simulink model "dss_2in_2048ch_983mhz_real"  (R2021a) (continued)
== system dss_2in_2048ch_983mhz_real == (continued)
      mask DialogControl t230_QT_dac3_AnalogSettings Prompt: Analog Settings
      mask DialogControl t230_DualTileConfig Prompt: Dual Tile Configuration
      mask DialogControl t230_DT_dac0 Prompt: DAC 0
      mask DialogControl t230_DT_dac0_DataSettings Prompt: Data Settings
      mask DialogControl t230_DT_dac0_req_axis_clk_label Prompt: Required AXI4-Stream clock:
      mask DialogControl t230_DT_dac0_MixerSettings Prompt: MIxer Settings
      mask DialogControl t230_DT_dac0_AnalogSettings Prompt: Analog Settings
      mask DialogControl t230_DT_dac1 Prompt: DAC 1
      mask DialogControl t230_DT_dac1_DataSettings Prompt: Data Settings
      mask DialogControl t230_DT_dac1_req_axis_clk_label Prompt: Required AXI4-Stream clock:
      mask DialogControl t230_DT_dac1_MixerSettings Prompt: MIxer Settings
      mask DialogControl t230_DT_dac1_AnalogSettings Prompt: Analog Settings
      mask DialogControl Tile231_container Prompt: Tile 231
      mask DialogControl pll_clocking_config_231 Prompt: Clocking and PLL Configuration
      mask DialogControl t231_QuadTileConfig Prompt: Quad Tile Configuration
      mask DialogControl t231_DACPair01 Prompt: DAC Pair 0,1
      mask DialogControl t231_QT_dac0 Prompt: DAC 0
      mask DialogControl t231_QT_dac0_DataSettings Prompt: Data Settings
      mask DialogControl t231_QT_dac0_req_axis_clk_label Prompt: Required AXI4-Stream clock:
      mask DialogControl t231_QT_dac0_MixerSettings Prompt: MIxer Settings
      mask DialogControl t231_QT_dac0_AnalogSettings Prompt: Analog Settings
      mask DialogControl t231_QT_dac1 Prompt: DAC 1
      mask DialogControl t231_QT_dac1_DataSettings Prompt: Data Settings
      mask DialogControl t231_QT_dac1_req_axis_clk_label Prompt: Required AXI4-Stream clock:
      mask DialogControl t231_QT_dac1_MixerSettings Prompt: MIxer Settings
      mask DialogControl t231_QT_dac1_AnalogSettings Prompt: Analog Settings
      mask DialogControl t231_DACPair23 Prompt: DAC Pair 2,3
      mask DialogControl t231_QT_dac2 Prompt: DAC 2
      mask DialogControl t231_QT_dac2_DataSettings Prompt: Data Settings
      mask DialogControl t231_QT_dac2_req_axis_clk_label Prompt: Required AXI4-Stream clock:
      mask DialogControl t231_QT_dac2_MixerSettings Prompt: MIxer Settings
      mask DialogControl t231_QT_dac2_AnalogSettings Prompt: Analog Settings
      mask DialogControl t231_QT_dac3 Prompt: DAC 3
      mask DialogControl t231_QT_dac3_DataSettings Prompt: Data Settings
      mask DialogControl t231_QT_dac3_req_axis_clk_label Prompt: Required AXI4-Stream clock:
      mask DialogControl t231_QT_dac3_MixerSettings Prompt: MIxer Settings
      mask DialogControl t231_QT_dac3_AnalogSettings Prompt: Analog Settings
      mask DialogControl t231_DualTileConfig Prompt: Dual Tile Configuration
      mask DialogControl t231_DT_dac0 Prompt: DAC 0
      mask DialogControl t231_DT_dac0_DataSettings Prompt: Data Settings
      mask DialogControl t231_DT_dac0_req_axis_clk_label Prompt: Required AXI4-Stream clock:
      mask DialogControl t231_DT_dac0_MixerSettings Prompt: MIxer Settings
      mask DialogControl t231_DT_dac0_AnalogSettings Prompt: Analog Settings
      mask DialogControl t231_DT_dac1 Prompt: DAC 1
      mask DialogControl t231_DT_dac1_DataSettings Prompt: Data Settings
      mask DialogControl t231_DT_dac1_req_axis_clk_label Prompt: Required AXI4-Stream clock:
      mask DialogControl t231_DT_dac1_MixerSettings Prompt: MIxer Settings
      mask DialogControl t231_DT_dac1_AnalogSettings Prompt: Analog Settings
      mask DialogControl SystemClocking Prompt: System Clocking
      mask DialogControl ADCClocking Prompt: ADC Tile Clocking
      mask DialogControl t224_clock_config Prompt: ADC Tile 224
      mask DialogControl t225_clock_config Prompt: ADC Tile 225
      mask DialogControl t226_clock_config Prompt: ADC Tile 226
      mask DialogControl t227_clock_config Prompt: ADC Tile 227
      mask DialogControl DACClocking Prompt: DAC Tile Clocking
      mask DialogControl t228_clock_config Prompt: DAC Tile 228
      mask DialogControl t229_clock_config Prompt: DAC Tile 229
      mask DialogControl t230_clock_config Prompt: DAC Tile 230
      mask DialogControl t231_clock_config Prompt: DAC Tile 231
  simple_bram_vacc : Subsystem  AncestorBlock=casper_library_accumulators/simple_bram_vacc  mask(simple_bram_vacc){vec_len=512, arith_type=Unsigned, n_bits=64, bin_pt=34}
      mask prompts: vec_len "Vector length:"; arith_type "Output type:"; n_bits "Bit width (output):"; bin_pt "Binary point (output):"
      mask help: This simple vector accumulator is based on a shift register. It outputs its previous accumulated vector after a new_acc pulse is received.
      mask init:
      | simple_bram_vacc_init(gcb, ...
      |     'vec_len', vec_len, ...
      |     'arith_type', arith_type, ...
      |     'n_bits', n_bits, ...
      |     'bin_pt', bin_pt);
      mask TypeOptions arith_type: Signed | Unsigned
  simple_bram_vacc1 : Subsystem  AncestorBlock=casper_library_accumulators/simple_bram_vacc  mask(simple_bram_vacc){vec_len=512, arith_type=Unsigned, n_bits=64, bin_pt=34}
      mask prompts: vec_len "Vector length:"; arith_type "Output type:"; n_bits "Bit width (output):"; bin_pt "Binary point (output):"
      mask help: This simple vector accumulator is based on a shift register. It outputs its previous accumulated vector after a new_acc pulse is received.
      mask init:
      | simple_bram_vacc_init(gcb, ...
      |     'vec_len', vec_len, ...
      |     'arith_type', arith_type, ...
      |     'n_bits', n_bits, ...
      |     'bin_pt', bin_pt);
      mask TypeOptions arith_type: Signed | Unsigned
  simple_bram_vacc10 : Subsystem  AncestorBlock=casper_library_accumulators/simple_bram_vacc  mask(simple_bram_vacc){vec_len=512, arith_type=Signed, n_bits=64, bin_pt=34}
      mask prompts: vec_len "Vector length:"; arith_type "Output type:"; n_bits "Bit width (output):"; bin_pt "Binary point (output):"
      mask help: This simple vector accumulator is based on a shift register. It outputs its previous accumulated vector after a new_acc pulse is received.
      mask init:
      | simple_bram_vacc_init(gcb, ...
      |     'vec_len', vec_len, ...
      |     'arith_type', arith_type, ...
      |     'n_bits', n_bits, ...
      |     'bin_pt', bin_pt);
      mask TypeOptions arith_type: Signed | Unsigned
  simple_bram_vacc11 : Subsystem  AncestorBlock=casper_library_accumulators/simple_bram_vacc  mask(simple_bram_vacc){vec_len=512, arith_type=Signed, n_bits=64, bin_pt=34}
      mask prompts: vec_len "Vector length:"; arith_type "Output type:"; n_bits "Bit width (output):"; bin_pt "Binary point (output):"
      mask help: This simple vector accumulator is based on a shift register. It outputs its previous accumulated vector after a new_acc pulse is received.
      mask init:
      | simple_bram_vacc_init(gcb, ...
      |     'vec_len', vec_len, ...
      |     'arith_type', arith_type, ...
      |     'n_bits', n_bits, ...
      |     'bin_pt', bin_pt);
      mask TypeOptions arith_type: Signed | Unsigned
  simple_bram_vacc12 : Subsystem  AncestorBlock=casper_library_accumulators/simple_bram_vacc  mask(simple_bram_vacc){vec_len=512, arith_type=Signed, n_bits=64, bin_pt=34}
      mask prompts: vec_len "Vector length:"; arith_type "Output type:"; n_bits "Bit width (output):"; bin_pt "Binary point (output):"
      mask help: This simple vector accumulator is based on a shift register. It outputs its previous accumulated vector after a new_acc pulse is received.
      mask init:
      | simple_bram_vacc_init(gcb, ...
      |     'vec_len', vec_len, ...
      |     'arith_type', arith_type, ...
      |     'n_bits', n_bits, ...
      |     'bin_pt', bin_pt);
      mask TypeOptions arith_type: Signed | Unsigned
  simple_bram_vacc13 : Subsystem  AncestorBlock=casper_library_accumulators/simple_bram_vacc  mask(simple_bram_vacc){vec_len=512, arith_type=Signed, n_bits=64, bin_pt=34}
      mask prompts: vec_len "Vector length:"; arith_type "Output type:"; n_bits "Bit width (output):"; bin_pt "Binary point (output):"
      mask help: This simple vector accumulator is based on a shift register. It outputs its previous accumulated vector after a new_acc pulse is received.
      mask init:
      | simple_bram_vacc_init(gcb, ...
      |     'vec_len', vec_len, ...
      |     'arith_type', arith_type, ...
      |     'n_bits', n_bits, ...
      |     'bin_pt', bin_pt);
      mask TypeOptions arith_type: Signed | Unsigned
  simple_bram_vacc14 : Subsystem  AncestorBlock=casper_library_accumulators/simple_bram_vacc  mask(simple_bram_vacc){vec_len=512, arith_type=Signed, n_bits=64, bin_pt=34}
      mask prompts: vec_len "Vector length:"; arith_type "Output type:"; n_bits "Bit width (output):"; bin_pt "Binary point (output):"
      mask help: This simple vector accumulator is based on a shift register. It outputs its previous accumulated vector after a new_acc pulse is received.
      mask init:
      | simple_bram_vacc_init(gcb, ...
      |     'vec_len', vec_len, ...
      |     'arith_type', arith_type, ...
      |     'n_bits', n_bits, ...
      |     'bin_pt', bin_pt);
      mask TypeOptions arith_type: Signed | Unsigned
  simple_bram_vacc15 : Subsystem  AncestorBlock=casper_library_accumulators/simple_bram_vacc  mask(simple_bram_vacc){vec_len=512, arith_type=Signed, n_bits=64, bin_pt=34}
      mask prompts: vec_len "Vector length:"; arith_type "Output type:"; n_bits "Bit width (output):"; bin_pt "Binary point (output):"
      mask help: This simple vector accumulator is based on a shift register. It outputs its previous accumulated vector after a new_acc pulse is received.
      mask init:
      | simple_bram_vacc_init(gcb, ...
      |     'vec_len', vec_len, ...
      |     'arith_type', arith_type, ...
      |     'n_bits', n_bits, ...
      |     'bin_pt', bin_pt);
      mask TypeOptions arith_type: Signed | Unsigned
  simple_bram_vacc16 : Subsystem  AncestorBlock=casper_library_accumulators/simple_bram_vacc  mask(simple_bram_vacc){vec_len=512, arith_type=Unsigned, n_bits=64, bin_pt=34}
      mask prompts: vec_len "Vector length:"; arith_type "Output type:"; n_bits "Bit width (output):"; bin_pt "Binary point (output):"
      mask help: This simple vector accumulator is based on a shift register. It outputs its previous accumulated vector after a new_acc pulse is received.
      mask init:
      | simple_bram_vacc_init(gcb, ...
      |     'vec_len', vec_len, ...
      |     'arith_type', arith_type, ...
      |     'n_bits', n_bits, ...
      |     'bin_pt', bin_pt);
      mask TypeOptions arith_type: Signed | Unsigned
  simple_bram_vacc17 : Subsystem  AncestorBlock=casper_library_accumulators/simple_bram_vacc  mask(simple_bram_vacc){vec_len=512, arith_type=Unsigned, n_bits=64, bin_pt=34}
      mask prompts: vec_len "Vector length:"; arith_type "Output type:"; n_bits "Bit width (output):"; bin_pt "Binary point (output):"
      mask help: This simple vector accumulator is based on a shift register. It outputs its previous accumulated vector after a new_acc pulse is received.
      mask init:
      | simple_bram_vacc_init(gcb, ...
      |     'vec_len', vec_len, ...
      |     'arith_type', arith_type, ...
      |     'n_bits', n_bits, ...
      |     'bin_pt', bin_pt);
      mask TypeOptions arith_type: Signed | Unsigned
  simple_bram_vacc18 : Subsystem  AncestorBlock=casper_library_accumulators/simple_bram_vacc  mask(simple_bram_vacc){vec_len=512, arith_type=Unsigned, n_bits=64, bin_pt=34}
      mask prompts: vec_len "Vector length:"; arith_type "Output type:"; n_bits "Bit width (output):"; bin_pt "Binary point (output):"
      mask help: This simple vector accumulator is based on a shift register. It outputs its previous accumulated vector after a new_acc pulse is received.
      mask init:
      | simple_bram_vacc_init(gcb, ...
      |     'vec_len', vec_len, ...
      |     'arith_type', arith_type, ...
      |     'n_bits', n_bits, ...
      |     'bin_pt', bin_pt);
      mask TypeOptions arith_type: Signed | Unsigned
  simple_bram_vacc19 : Subsystem  AncestorBlock=casper_library_accumulators/simple_bram_vacc  mask(simple_bram_vacc){vec_len=512, arith_type=Unsigned, n_bits=64, bin_pt=34}
      mask prompts: vec_len "Vector length:"; arith_type "Output type:"; n_bits "Bit width (output):"; bin_pt "Binary point (output):"
      mask help: This simple vector accumulator is based on a shift register. It outputs its previous accumulated vector after a new_acc pulse is received.
      mask init:
      | simple_bram_vacc_init(gcb, ...
      |     'vec_len', vec_len, ...
      |     'arith_type', arith_type, ...
      |     'n_bits', n_bits, ...
      |     'bin_pt', bin_pt);
      mask TypeOptions arith_type: Signed | Unsigned
  simple_bram_vacc2 : Subsystem  AncestorBlock=casper_library_accumulators/simple_bram_vacc  mask(simple_bram_vacc){vec_len=512, arith_type=Unsigned, n_bits=64, bin_pt=34}
      mask prompts: vec_len "Vector length:"; arith_type "Output type:"; n_bits "Bit width (output):"; bin_pt "Binary point (output):"
      mask help: This simple vector accumulator is based on a shift register. It outputs its previous accumulated vector after a new_acc pulse is received.
      mask init:
      | simple_bram_vacc_init(gcb, ...
      |     'vec_len', vec_len, ...
      |     'arith_type', arith_type, ...
      |     'n_bits', n_bits, ...
      |     'bin_pt', bin_pt);
      mask TypeOptions arith_type: Signed | Unsigned
  simple_bram_vacc20 : Subsystem  AncestorBlock=casper_library_accumulators/simple_bram_vacc  mask(simple_bram_vacc){vec_len=512, arith_type=Unsigned, n_bits=64, bin_pt=34}
      mask prompts: vec_len "Vector length:"; arith_type "Output type:"; n_bits "Bit width (output):"; bin_pt "Binary point (output):"
      mask help: This simple vector accumulator is based on a shift register. It outputs its previous accumulated vector after a new_acc pulse is received.
      mask init:
      | simple_bram_vacc_init(gcb, ...
      |     'vec_len', vec_len, ...
      |     'arith_type', arith_type, ...
      |     'n_bits', n_bits, ...
      |     'bin_pt', bin_pt);
      mask TypeOptions arith_type: Signed | Unsigned
  simple_bram_vacc21 : Subsystem  AncestorBlock=casper_library_accumulators/simple_bram_vacc  mask(simple_bram_vacc){vec_len=512, arith_type=Unsigned, n_bits=64, bin_pt=34}
      mask prompts: vec_len "Vector length:"; arith_type "Output type:"; n_bits "Bit width (output):"; bin_pt "Binary point (output):"
      mask help: This simple vector accumulator is based on a shift register. It outputs its previous accumulated vector after a new_acc pulse is received.
      mask init:
      | simple_bram_vacc_init(gcb, ...
      |     'vec_len', vec_len, ...
      |     'arith_type', arith_type, ...
      |     'n_bits', n_bits, ...
      |     'bin_pt', bin_pt);
      mask TypeOptions arith_type: Signed | Unsigned
  simple_bram_vacc22 : Subsystem  AncestorBlock=casper_library_accumulators/simple_bram_vacc  mask(simple_bram_vacc){vec_len=512, arith_type=Unsigned, n_bits=64, bin_pt=34}
      mask prompts: vec_len "Vector length:"; arith_type "Output type:"; n_bits "Bit width (output):"; bin_pt "Binary point (output):"
      mask help: This simple vector accumulator is based on a shift register. It outputs its previous accumulated vector after a new_acc pulse is received.
      mask init:
      | simple_bram_vacc_init(gcb, ...
      |     'vec_len', vec_len, ...
      |     'arith_type', arith_type, ...
      |     'n_bits', n_bits, ...
      |     'bin_pt', bin_pt);
      mask TypeOptions arith_type: Signed | Unsigned
  simple_bram_vacc23 : Subsystem  AncestorBlock=casper_library_accumulators/simple_bram_vacc  mask(simple_bram_vacc){vec_len=512, arith_type=Unsigned, n_bits=64, bin_pt=34}
      mask prompts: vec_len "Vector length:"; arith_type "Output type:"; n_bits "Bit width (output):"; bin_pt "Binary point (output):"
      mask help: This simple vector accumulator is based on a shift register. It outputs its previous accumulated vector after a new_acc pulse is received.
      mask init:
      | simple_bram_vacc_init(gcb, ...
      |     'vec_len', vec_len, ...
      |     'arith_type', arith_type, ...
      |     'n_bits', n_bits, ...
      |     'bin_pt', bin_pt);
      mask TypeOptions arith_type: Signed | Unsigned
  simple_bram_vacc3 : Subsystem  AncestorBlock=casper_library_accumulators/simple_bram_vacc  mask(simple_bram_vacc){vec_len=512, arith_type=Unsigned, n_bits=64, bin_pt=34}
      mask prompts: vec_len "Vector length:"; arith_type "Output type:"; n_bits "Bit width (output):"; bin_pt "Binary point (output):"
      mask help: This simple vector accumulator is based on a shift register. It outputs its previous accumulated vector after a new_acc pulse is received.
      mask init:
      | simple_bram_vacc_init(gcb, ...
      |     'vec_len', vec_len, ...
      |     'arith_type', arith_type, ...
      |     'n_bits', n_bits, ...
      |     'bin_pt', bin_pt);
      mask TypeOptions arith_type: Signed | Unsigned
  simple_bram_vacc4 : Subsystem  AncestorBlock=casper_library_accumulators/simple_bram_vacc  mask(simple_bram_vacc){vec_len=512, arith_type=Unsigned, n_bits=64, bin_pt=34}
      mask prompts: vec_len "Vector length:"; arith_type "Output type:"; n_bits "Bit width (output):"; bin_pt "Binary point (output):"
      mask help: This simple vector accumulator is based on a shift register. It outputs its previous accumulated vector after a new_acc pulse is received.
      mask init:
      | simple_bram_vacc_init(gcb, ...
      |     'vec_len', vec_len, ...
      |     'arith_type', arith_type, ...
      |     'n_bits', n_bits, ...
      |     'bin_pt', bin_pt);
      mask TypeOptions arith_type: Signed | Unsigned
  simple_bram_vacc5 : Subsystem  AncestorBlock=casper_library_accumulators/simple_bram_vacc  mask(simple_bram_vacc){vec_len=512, arith_type=Unsigned, n_bits=64, bin_pt=34}
      mask prompts: vec_len "Vector length:"; arith_type "Output type:"; n_bits "Bit width (output):"; bin_pt "Binary point (output):"
      mask help: This simple vector accumulator is based on a shift register. It outputs its previous accumulated vector after a new_acc pulse is received.
      mask init:
      | simple_bram_vacc_init(gcb, ...
      |     'vec_len', vec_len, ...
      |     'arith_type', arith_type, ...
      |     'n_bits', n_bits, ...
      |     'bin_pt', bin_pt);
      mask TypeOptions arith_type: Signed | Unsigned
  simple_bram_vacc6 : Subsystem  AncestorBlock=casper_library_accumulators/simple_bram_vacc  mask(simple_bram_vacc){vec_len=512, arith_type=Unsigned, n_bits=64, bin_pt=34}
      mask prompts: vec_len "Vector length:"; arith_type "Output type:"; n_bits "Bit width (output):"; bin_pt "Binary point (output):"
      mask help: This simple vector accumulator is based on a shift register. It outputs its previous accumulated vector after a new_acc pulse is received.
      mask init:
      | simple_bram_vacc_init(gcb, ...
      |     'vec_len', vec_len, ...
      |     'arith_type', arith_type, ...
      |     'n_bits', n_bits, ...
      |     'bin_pt', bin_pt);
      mask TypeOptions arith_type: Signed | Unsigned
  simple_bram_vacc7 : Subsystem  AncestorBlock=casper_library_accumulators/simple_bram_vacc  mask(simple_bram_vacc){vec_len=512, arith_type=Unsigned, n_bits=64, bin_pt=34}
      mask prompts: vec_len "Vector length:"; arith_type "Output type:"; n_bits "Bit width (output):"; bin_pt "Binary point (output):"
      mask help: This simple vector accumulator is based on a shift register. It outputs its previous accumulated vector after a new_acc pulse is received.
      mask init:
      | simple_bram_vacc_init(gcb, ...
      |     'vec_len', vec_len, ...
      |     'arith_type', arith_type, ...
      |     'n_bits', n_bits, ...
      |     'bin_pt', bin_pt);
      mask TypeOptions arith_type: Signed | Unsigned
  simple_bram_vacc8 : Subsystem  AncestorBlock=casper_library_accumulators/simple_bram_vacc  mask(simple_bram_vacc){vec_len=512, arith_type=Signed, n_bits=64, bin_pt=34}
      mask prompts: vec_len "Vector length:"; arith_type "Output type:"; n_bits "Bit width (output):"; bin_pt "Binary point (output):"
      mask help: This simple vector accumulator is based on a shift register. It outputs its previous accumulated vector after a new_acc pulse is received.
      mask init:
      | simple_bram_vacc_init(gcb, ...
      |     'vec_len', vec_len, ...
      |     'arith_type', arith_type, ...
      |     'n_bits', n_bits, ...
      |     'bin_pt', bin_pt);
      mask TypeOptions arith_type: Signed | Unsigned
  simple_bram_vacc9 : Subsystem  AncestorBlock=casper_library_accumulators/simple_bram_vacc  mask(simple_bram_vacc){vec_len=512, arith_type=Signed, n_bits=64, bin_pt=34}
      mask prompts: vec_len "Vector length:"; arith_type "Output type:"; n_bits "Bit width (output):"; bin_pt "Binary point (output):"
      mask help: This simple vector accumulator is based on a shift register. It outputs its previous accumulated vector after a new_acc pulse is received.
      mask init:
      | simple_bram_vacc_init(gcb, ...
      |     'vec_len', vec_len, ...
      |     'arith_type', arith_type, ...
      |     'n_bits', n_bits, ...
      |     'bin_pt', bin_pt);
      mask TypeOptions arith_type: Signed | Unsigned
  spect_cnt{,1..3} (4 alike) : xbsIndex_r4/Counter  cnt_type=Free Running  cnt_to=Inf  operation=Up  start_count=0  cnt_by_val=1  arith_type=Unsigned  n_bits=9  bin_pt=0  load_pin=off  rst=on  en=on  explicit_period=on  period=1  use_behavioral_HDL=off  implementation=Fabric  use_rpm=off  dbl_ovrd=off  block_type=counter
  sync_gen : Subsystem  AncestorBlock=casper_library_misc/sync_gen  mask(sync_gen){sim_acc_len=1, fft_size=4096, fft_simult_inputs=8, pfb_fir_taps=4, reorder_vec=[2  2  9], scale=1}
      mask prompts: sim_acc_len "Simulation Accumulation Length"; fft_size "FFT Size"; fft_simult_inputs "Simultaneous Inputs (FFT)"; pfb_fir_taps "Taps in pfb_fir (use 1 if no pfb_fir)"; reorder_vec "Reorder Orders (e.g. [2 2 2])"
      mask help:
      | This block computes the sync period based on system parameters as
      | described in Casper memo.  Detailed descritions of the block's
      | variables can be found in the 'Block Documentation' section of
      | the CASPER wiki.
      mask init:
      | sync_gen_init(gcb, ...
      |     'sim_acc_len', sim_acc_len, ...
      |     'fft_size', fft_size, ...
      |     'fft_simult_inputs', fft_simult_inputs,...
      |     'pfb_fir_taps', pfb_fir_taps, ...
      |     'reorder_vec', reorder_vec, ...
      |     'scale', scale);
      settings: AttributesFormatString=sim_sync_period=36864
      mask Help:
      | SyncPeriod=LCM(reorder_vec)*(acc_num)*(pfb_fir_len)*(fft_size/fft_simult_inputs)
      | 
      | See CASPER sync pulse memo for more details.
  synth0_0 : Subsystem  Tag=xps:bram  AncestorBlock=xps_library/Memory/shared_bram  mask{arith_type=Unsigned, addr_width=9, data_width=64, reg_prim_output=on, reg_core_output=on, optimization=Minimum_Area, data_bin_pt=0, init_vals=[0:2^9-1], sample_rate=1}
      mask prompts: arith_type "Output Data Type"; addr_width "Address width"; data_width "Data Width"; reg_prim_output "Register Primitive Output"; reg_core_output "Register Core Output"; data_bin_pt "Data Binary Point"; init_vals "Initial values (simulation only)"; sample_rate "Sample rate"
      mask init:
      | shared_bram_mask;
      mask TypeOptions arith_type: Unsigned | Signed  (2's comp)
      mask TypeOptions data_width: 8 | 16 | 32 | 64 | 128
      mask TypeOptions optimization: Minimum_Area | Low_Power
  synth0_1 : Subsystem  Tag=xps:bram  AncestorBlock=xps_library/Memory/shared_bram  mask{arith_type=Unsigned, addr_width=9, data_width=64, reg_prim_output=on, reg_core_output=on, optimization=Minimum_Area, data_bin_pt=0, init_vals=[0:2^9-1], sample_rate=1}
      mask prompts: arith_type "Output Data Type"; addr_width "Address width"; data_width "Data Width"; reg_prim_output "Register Primitive Output"; reg_core_output "Register Core Output"; data_bin_pt "Data Binary Point"; init_vals "Initial values (simulation only)"; sample_rate "Sample rate"
      mask init:
      | shared_bram_mask;
      mask TypeOptions arith_type: Unsigned | Signed  (2's comp)
      mask TypeOptions data_width: 8 | 16 | 32 | 64 | 128
      mask TypeOptions optimization: Minimum_Area | Low_Power
  synth0_2 : Subsystem  Tag=xps:bram  AncestorBlock=xps_library/Memory/shared_bram  mask{arith_type=Unsigned, addr_width=9, data_width=64, reg_prim_output=on, reg_core_output=on, optimization=Minimum_Area, data_bin_pt=0, init_vals=[0:2^9-1], sample_rate=1}
      mask prompts: arith_type "Output Data Type"; addr_width "Address width"; data_width "Data Width"; reg_prim_output "Register Primitive Output"; reg_core_output "Register Core Output"; data_bin_pt "Data Binary Point"; init_vals "Initial values (simulation only)"; sample_rate "Sample rate"
      mask init:
      | shared_bram_mask;
      mask TypeOptions arith_type: Unsigned | Signed  (2's comp)
      mask TypeOptions data_width: 8 | 16 | 32 | 64 | 128
      mask TypeOptions optimization: Minimum_Area | Low_Power
  synth0_3 : Subsystem  Tag=xps:bram  AncestorBlock=xps_library/Memory/shared_bram  mask{arith_type=Unsigned, addr_width=9, data_width=64, reg_prim_output=on, reg_core_output=on, optimization=Minimum_Area, data_bin_pt=0, init_vals=[0:2^9-1], sample_rate=1}
      mask prompts: arith_type "Output Data Type"; addr_width "Address width"; data_width "Data Width"; reg_prim_output "Register Primitive Output"; reg_core_output "Register Core Output"; data_bin_pt "Data Binary Point"; init_vals "Initial values (simulation only)"; sample_rate "Sample rate"
      mask init:
      | shared_bram_mask;
      mask TypeOptions arith_type: Unsigned | Signed  (2's comp)
      mask TypeOptions data_width: 8 | 16 | 32 | 64 | 128
      mask TypeOptions optimization: Minimum_Area | Low_Power
  synth1_0 : Subsystem  Tag=xps:bram  AncestorBlock=xps_library/Memory/shared_bram  mask{arith_type=Unsigned, addr_width=9, data_width=64, reg_prim_output=on, reg_core_output=on, optimization=Minimum_Area, data_bin_pt=0, init_vals=[0:2^9-1], sample_rate=1}
      mask prompts: arith_type "Output Data Type"; addr_width "Address width"; data_width "Data Width"; reg_prim_output "Register Primitive Output"; reg_core_output "Register Core Output"; data_bin_pt "Data Binary Point"; init_vals "Initial values (simulation only)"; sample_rate "Sample rate"
      mask init:
      | shared_bram_mask;
      mask TypeOptions arith_type: Unsigned | Signed  (2's comp)
      mask TypeOptions data_width: 8 | 16 | 32 | 64 | 128
      mask TypeOptions optimization: Minimum_Area | Low_Power
  synth1_1 : Subsystem  Tag=xps:bram  AncestorBlock=xps_library/Memory/shared_bram  mask{arith_type=Unsigned, addr_width=9, data_width=64, reg_prim_output=on, reg_core_output=on, optimization=Minimum_Area, data_bin_pt=0, init_vals=[0:2^9-1], sample_rate=1}
      mask prompts: arith_type "Output Data Type"; addr_width "Address width"; data_width "Data Width"; reg_prim_output "Register Primitive Output"; reg_core_output "Register Core Output"; data_bin_pt "Data Binary Point"; init_vals "Initial values (simulation only)"; sample_rate "Sample rate"
      mask init:
      | shared_bram_mask;
      mask TypeOptions arith_type: Unsigned | Signed  (2's comp)
      mask TypeOptions data_width: 8 | 16 | 32 | 64 | 128
      mask TypeOptions optimization: Minimum_Area | Low_Power
  synth1_2 : Subsystem  Tag=xps:bram  AncestorBlock=xps_library/Memory/shared_bram  mask{arith_type=Unsigned, addr_width=9, data_width=64, reg_prim_output=on, reg_core_output=on, optimization=Minimum_Area, data_bin_pt=0, init_vals=[0:2^9-1], sample_rate=1}
      mask prompts: arith_type "Output Data Type"; addr_width "Address width"; data_width "Data Width"; reg_prim_output "Register Primitive Output"; reg_core_output "Register Core Output"; data_bin_pt "Data Binary Point"; init_vals "Initial values (simulation only)"; sample_rate "Sample rate"
      mask init:
      | shared_bram_mask;
      mask TypeOptions arith_type: Unsigned | Signed  (2's comp)
      mask TypeOptions data_width: 8 | 16 | 32 | 64 | 128
      mask TypeOptions optimization: Minimum_Area | Low_Power
  synth1_3 : Subsystem  Tag=xps:bram  AncestorBlock=xps_library/Memory/shared_bram  mask{arith_type=Unsigned, addr_width=9, data_width=64, reg_prim_output=on, reg_core_output=on, optimization=Minimum_Area, data_bin_pt=0, init_vals=[0:2^9-1], sample_rate=1}
      mask prompts: arith_type "Output Data Type"; addr_width "Address width"; data_width "Data Width"; reg_prim_output "Register Primitive Output"; reg_core_output "Register Core Output"; data_bin_pt "Data Binary Point"; init_vals "Initial values (simulation only)"; sample_rate "Sample rate"
      mask init:
      | shared_bram_mask;
      mask TypeOptions arith_type: Unsigned | Signed  (2's comp)
      mask TypeOptions data_width: 8 | 16 | 32 | 64 | 128
      mask TypeOptions optimization: Minimum_Area | Low_Power
  write_brams : Subsystem  Tag=xps:sw_reg  AncestorBlock=xps_library/Memory/software_register  mask(swreg){io_dir=From Processor, io_delay=0, init_val=0, sample_period=1, names=reg, bitwidths=32, bin_pts=0, arith_types=2, sim_port=on, show_format=off}
      mask prompts: io_dir "I/O direction"; io_delay "I/O delay"; init_val "Initial Value"; sample_period "Sample period"; names "Bitfield names [msb...lsb]"; bitwidths "Bitfield widths"; bin_pts "Bitfield binary pts"; arith_types "Bitfield types, ufix=0, fix=1, bool=2"; sim_port "Provide sim input/output?"; show_format "Print format string?"
      mask help: A 32-bit software-accessible register. Can be divided into bitfields of varying widths and types using the fields in the block mask.
      mask init:
      | swreg_init(gcb)
      settings: AttributesFormatString=1input
      mask TypeOptions io_dir: To Processor | From Processor
      mask Callback io_dir:
      | mask_enables = get_param(gcb, 'MaskEnables');
      | mask_names = get_param(gcb, 'MaskNames');
      | if strcmp(get_param(gcb, 'io_dir'), 'To Processor'),
      |     mask_enables{ismember(mask_names, 'sample_period')} = 'off';
      | else
      |     mask_enables{ismember(mask_names, 'sample_period')} = 'on';
      | end
      | set_param(gcb, 'MaskEnables', mask_enables);
      mask DialogControl ParameterTabVar0 Prompt: Setup
  tag scope: write_brams=global
  wires:
  Constant/1 -> adc_snapshot0/3(trig), adc_snapshot0/2(we)
  pipeline11/1(q) -> spect_cnt/1
  a2_1/1(data_out) -> Terminator14/1
  power4/1 -> Delay8/1
  fft_wideband_real/2(out00) -> Delay15/1
  Delay10/1 -> bram_mult0_3/1(In1), bram_mult0_2/1(In1), bram_mult0_1/1(In1), bram_mult0_0/1(In1), pipeline162/1(d), Delay34/1
  rfdc/1(m00_axis_tdata) -> munge/1(din), Reinterpret9/1
  fft_wideband_real/6(of) -> Terminator/1
  simple_bram_vacc/1(dout) -> Reinterpret4/1
  a2_0/1(data_out) -> Terminator13/1
  Reinterpret4/1 -> pipeline24/1(d)
  Constant19/1 -> cnt_rst/1(sim_1)
  a2_3/1(data_out) -> Terminator3/1
  bus_expand/7(out2) -> pfb_fir_real/8(pol1_in7)
  Delay15/1 -> Delay5/1
  bus_expand/5(out4) -> pfb_fir_real/6(pol1_in5)
  brams_control/1(we) -> a2_0/3(we)
  fft_wideband_real/1(sync_out) -> Delay14/1
  Delay6/1 -> Delay7/1
  fft_wideband_real/3(out01) -> Delay16/1
  pipeline24/1(q) -> a2_0/2(data_in)
  bus_expand/4(out5) -> pfb_fir_real/5(pol1_in4)
  bus_expand/3(out6) -> pfb_fir_real/4(pol1_in3)
  fft_wideband_real/4(out02) -> Delay2/1
  Delay14/1 -> Delay10/1
  pfb_fir_real/9(pol1_out8) -> fft_wideband_real/10(in07)
  pfb_fir_real/3(pol1_out2) -> fft_wideband_real/4(in01)
  pfb_fir_real/6(pol1_out5) -> fft_wideband_real/7(in04)
  power3/1 -> Delay4/1
  power2/1 -> Delay26/1
  bus_expand/2(out7) -> pfb_fir_real/3(pol1_in2)
  Constant16/1 -> acc_len/1(sim_1)
  simple_bram_vacc/2(valid) -> spect_cnt/2, brams_control/1(valid)
  simple_bram_vacc2/1(dout) -> Reinterpret1/1
  pfb_fir_real/2(pol1_out1) -> fft_wideband_real/3(in00)
  Delay24/1 -> acc_cntrl/2(sync)
  pfb_fir_real/5(pol1_out4) -> fft_wideband_real/6(in03)
  sync_gen/1(sync_out) -> Delay23/1
  pipeline8/1(q) -> [acc_len]
  bus_expand/1(msb_out8) -> pfb_fir_real/2(pol1_in1)
  bus_expand/8(lsb_out1) -> pfb_fir_real/9(pol1_in8)
  Delay16/1 -> Delay17/1
  a2_2/1(data_out) -> Terminator2/1
  bus_expand/6(out3) -> pfb_fir_real/7(pol1_in6)
  pipeline9/1(q) -> [cnt_rst]
  Delay34/1 -> Delay24/1
  [cnt_rst] -> acc_cntrl/3(rst)
  Constant2/1 -> fft_wideband_real/2(shift)
  pfb_fir_real/8(pol1_out7) -> fft_wideband_real/9(in06)
  pipeline3/1(q) -> pfb_fir_real/1(sync)
  simple_bram_vacc3/1(dout) -> Reinterpret2/1
  pfb_fir_real/1(sync_out) -> fft_wideband_real/1(sync)
  cnt_rst/1(in_reg) -> pipeline9/1(d)
  Delay2/1 -> Delay3/1
  munge/1(dout) -> bus_expand/1(bus_in)
  power1/1 -> Delay25/1
  [acc_len] -> acc_cntrl/1(acc_len)
  acc_len/1(in_reg) -> pipeline8/1(d)
  pfb_fir_real/7(pol1_out6) -> fft_wideband_real/8(in05)
  simple_bram_vacc1/1(dout) -> Reinterpret5/1
  fft_wideband_real/5(out03) -> Delay6/1
  adc_snapshot0/1(arm_out) -> Terminator4/1
  pipeline1/1(q) -> a2_0/1(addr)
  pfb_fir_real/4(pol1_out3) -> fft_wideband_real/5(in02)
  Reinterpret9/1 -> adc_snapshot0/1(in_d)
  pipeline7/1(q) -> a2_1/1(addr)
  pipeline16/1(q) -> a2_1/2(data_in)
  brams_control1/1(we) -> a2_1/3(we)
  Reinterpret5/1 -> pipeline16/1(d)
  simple_bram_vacc1/2(valid) -> brams_control1/1(valid)
  Reinterpret1/1 -> pipeline17/1(d)
  pipeline17/1(q) -> a2_2/2(data_in)
  Reinterpret2/1 -> pipeline19/1(d)
  pipeline20/1(q) -> a2_3/1(addr)
  pipeline19/1(q) -> a2_3/2(data_in)
  brams_control3/1(we) -> a2_3/3(we)
  simple_bram_vacc3/2(valid) -> brams_control3/1(valid)
  simple_bram_vacc2/2(valid) -> brams_control2/1(valid)
  pipeline18/1(q) -> a2_2/1(addr)
  brams_control2/1(we) -> a2_2/3(we)
  pipeline5/1(q) -> simple_bram_vacc/1(new_acc)
  pipeline6/1(q) -> simple_bram_vacc/2(din)
  pipeline12/1(q) -> simple_bram_vacc1/1(new_acc)
  pipeline13/1(q) -> simple_bram_vacc1/2(din)
  pipeline22/1(q) -> simple_bram_vacc2/1(new_acc)
  pipeline27/1(q) -> simple_bram_vacc2/2(din)
  pipeline14/1(q) -> simple_bram_vacc3/1(new_acc)
  pipeline15/1(q) -> simple_bram_vacc3/2(din)
  acc_cntrl/1(new_acc) -> pipeline11/1(d), pipeline82/1(d), pipeline90/1(d), pipeline80/1(d), pipeline100/1(d), pipeline59/1(d), pipeline67/1(d), pipeline57/1(d), pipeline77/1(d), pipeline14/1(d), pipeline22/1(d), pipeline12/1(d), pipeline5/1(d)
  pipeline29/1(q) -> pipeline6/1(d)
  pipeline30/1(q) -> pipeline13/1(d)
  pipeline31/1(q) -> pipeline27/1(d)
  pipeline32/1(q) -> pipeline15/1(d)
  Delay25/1 -> pipeline29/1(d)
  Delay26/1 -> pipeline30/1(d)
  Delay4/1 -> pipeline31/1(d)
  Delay8/1 -> pipeline32/1(d)
  Delay33/1 -> pipeline51/1(d)
  Delay29/1 -> pipeline50/1(d)
  Delay22/1 -> pipeline49/1(d)
  Delay21/1 -> pipeline47/1(d)
  pipeline51/1(q) -> pipeline34/1(d)
  pipeline50/1(q) -> pipeline46/1(d)
  pipeline49/1(q) -> pipeline28/1(d)
  pipeline47/1(q) -> pipeline53/1(d)
  acc_cntrl1/1(new_acc) -> pipeline52/1(d), pipeline10/1(d), pipeline41/1(d), pipeline33/1(d), pipeline4/1(d)
  pipeline34/1(q) -> simple_bram_vacc7/2(din)
  pipeline33/1(q) -> simple_bram_vacc7/1(new_acc)
  pipeline46/1(q) -> simple_bram_vacc6/2(din)
  pipeline41/1(q) -> simple_bram_vacc6/1(new_acc)
  pipeline28/1(q) -> simple_bram_vacc5/2(din)
  pipeline10/1(q) -> simple_bram_vacc5/1(new_acc)
  pipeline53/1(q) -> simple_bram_vacc4/2(din)
  pipeline52/1(q) -> simple_bram_vacc4/1(new_acc)
  brams_control14/1(we) -> b2_2/3(we)
  pipeline37/1(q) -> b2_2/1(addr)
  simple_bram_vacc6/2(valid) -> brams_control14/1(valid)
  simple_bram_vacc7/2(valid) -> brams_control15/1(valid)
  brams_control15/1(we) -> b2_3/3(we)
  pipeline38/1(q) -> b2_3/2(data_in)
  pipeline39/1(q) -> b2_3/1(addr)
  Reinterpret6/1 -> pipeline38/1(d)
  pipeline36/1(q) -> b2_2/2(data_in)
  Reinterpret3/1 -> pipeline36/1(d)
  simple_bram_vacc5/2(valid) -> brams_control13/1(valid)
  Reinterpret8/1 -> pipeline35/1(d)
  brams_control13/1(we) -> b2_1/3(we)
  pipeline35/1(q) -> b2_1/2(data_in)
  pipeline42/1(q) -> b2_1/1(addr)
  Reinterpret10/1 -> adc_snapshot1/1(in_d)
  pfb_fir_real1/4(pol1_out3) -> fft_wideband_real1/5(in02)
  pipeline2/1(q) -> b2_0/1(addr)
  adc_snapshot1/1(arm_out) -> Terminator9/1
  fft_wideband_real1/5(out03) -> Delay31/1
  simple_bram_vacc5/1(dout) -> Reinterpret8/1
  pfb_fir_real1/7(pol1_out6) -> fft_wideband_real1/8(in05)
  Delay27/1 -> power7/1, cmult2/2(b), pipeline155/1(d), bram_mult0_2/2(d)
  power5/1 -> Delay21/1
  munge1/1(dout) -> bus_expand1/1(bus_in)
  Delay18/1 -> Delay27/1
  pfb_fir_real1/1(sync_out) -> fft_wideband_real1/1(sync)
  simple_bram_vacc7/1(dout) -> Reinterpret6/1
  pipeline48/1(q) -> pfb_fir_real1/1(sync)
  pfb_fir_real1/8(pol1_out7) -> fft_wideband_real1/9(in06)
  Constant3/1 -> fft_wideband_real1/2(shift)
  [cnt_rst] -> acc_cntrl1/3(rst)
  Delay28/1 -> Delay20/1
  bus_expand1/6(out3) -> pfb_fir_real1/7(pol1_in6)
  b2_2/1(data_out) -> Terminator7/1
  Delay12/1 -> Delay13/1
  bus_expand1/8(lsb_out1) -> pfb_fir_real1/9(pol1_in8)
  bus_expand1/1(msb_out8) -> pfb_fir_real1/2(pol1_in1)
  pfb_fir_real1/5(pol1_out4) -> fft_wideband_real1/6(in03)
  Delay20/1 -> acc_cntrl1/2(sync)
  pfb_fir_real1/2(pol1_out1) -> fft_wideband_real1/3(in00)
  simple_bram_vacc6/1(dout) -> Reinterpret3/1
  simple_bram_vacc4/2(valid) -> brams_control12/1(valid), spect_cnt1/2
  bus_expand1/2(out7) -> pfb_fir_real1/3(pol1_in2)
  power6/1 -> Delay22/1
  power7/1 -> Delay29/1
  pfb_fir_real1/6(pol1_out5) -> fft_wideband_real1/7(in04)
  pfb_fir_real1/3(pol1_out2) -> fft_wideband_real1/4(in01)
  pfb_fir_real1/9(pol1_out8) -> fft_wideband_real1/10(in07)
  Delay23/1 -> pipeline48/1(d), pipeline3/1(d)
  Delay9/1 -> Delay1/1
  fft_wideband_real1/4(out02) -> Delay18/1
  bus_expand1/3(out6) -> pfb_fir_real1/4(pol1_in3)
  bus_expand1/4(out5) -> pfb_fir_real1/5(pol1_in4)
  pipeline40/1(q) -> b2_0/2(data_in)
  fft_wideband_real1/3(out01) -> Delay12/1
  Delay31/1 -> Delay32/1
  fft_wideband_real1/1(sync_out) -> Delay9/1
  brams_control12/1(we) -> b2_0/3(we)
  bus_expand1/5(out4) -> pfb_fir_real1/6(pol1_in5)
  Delay11/1 -> Delay30/1
  Delay13/1 -> power6/1, cmult1/2(b), pipeline154/1(d), bram_mult0_1/2(d)
  bus_expand1/7(out2) -> pfb_fir_real1/8(pol1_in7)
  b2_3/1(data_out) -> Terminator8/1
  Reinterpret7/1 -> pipeline40/1(d)
  b2_0/1(data_out) -> Terminator5/1
  spect_cnt1/1 -> pipeline2/1(d), pipeline42/1(d), pipeline37/1(d), pipeline39/1(d)
  simple_bram_vacc4/1(dout) -> Reinterpret7/1
  Delay32/1 -> power8/1, cmult3/2(b), pipeline156/1(d), bram_mult0_3/2(d)
  Delay30/1 -> power5/1, pipeline153/1(d), bram_mult0_0/2(d), cmult/2(b)
  fft_wideband_real1/6(of) -> Terminator1/1
  Delay1/1 -> bram_mult1_0/1(In1), bram_mult1_1/1(In1), bram_mult1_2/1(In1), bram_mult1_3/1(In1), pipeline164/1(d), Delay28/1
  fft_wideband_real1/2(out00) -> Delay11/1
  power8/1 -> Delay33/1
  b2_1/1(data_out) -> Terminator6/1
  pipeline4/1(q) -> spect_cnt1/1
  Constant1/1 -> adc_snapshot1/2(we), adc_snapshot1/3(trig)
  rfdc/2(m02_axis_tdata) -> Reinterpret10/1, munge1/1(din)
  [acc_len] -> acc_cntrl1/1(acc_len)
  rfdc/3(m10_axis_tdata) -> Terminator10/1
  rfdc/4(m12_axis_tdata) -> Terminator11/1
  rfdc/6(m22_axis_tdata) -> Terminator15/1
  rfdc/5(m20_axis_tdata) -> Terminator12/1
  rfdc/8(m32_axis_tdata) -> Terminator17/1
  rfdc/7(m30_axis_tdata) -> Terminator16/1
  Band-Limited White Noise/1 -> rfdc/8(m32_axis_tdata_sim), rfdc/7(m30_axis_tdata_sim), rfdc/6(m22_axis_tdata_sim), rfdc/5(m20_axis_tdata_sim), rfdc/4(m12_axis_tdata_sim), rfdc/3(m10_axis_tdata_sim), rfdc/2(m02_axis_tdata_sim), rfdc/1(m00_axis_tdata_sim)
  Delay40/1 -> pipeline76/1(d)
  Delay39/1 -> pipeline75/1(d)
  Delay37/1 -> pipeline74/1(d)
  Delay36/1 -> pipeline73/1(d)
  pipeline76/1(q) -> pipeline60/1(d)
  pipeline75/1(q) -> pipeline72/1(d)
  pipeline74/1(q) -> pipeline58/1(d)
  pipeline73/1(q) -> pipeline78/1(d)
  pipeline60/1(q) -> simple_bram_vacc11/2(din)
  pipeline59/1(q) -> simple_bram_vacc11/1(new_acc)
  pipeline72/1(q) -> simple_bram_vacc10/2(din)
  pipeline67/1(q) -> simple_bram_vacc10/1(new_acc)
  pipeline58/1(q) -> simple_bram_vacc9/2(din)
  pipeline57/1(q) -> simple_bram_vacc9/1(new_acc)
  pipeline78/1(q) -> simple_bram_vacc8/2(din)
  pipeline77/1(q) -> simple_bram_vacc8/1(new_acc)
  brams_control6/1(we) -> ab_re1/3(we)
  pipeline26/1(q) -> ab_re1/1(addr)
  simple_bram_vacc10/2(valid) -> brams_control6/1(valid)
  simple_bram_vacc11/2(valid) -> brams_control7/1(valid)
  brams_control7/1(we) -> ab_im1/3(we)
  pipeline55/1(q) -> ab_im1/2(data_in)
  pipeline56/1(q) -> ab_im1/1(addr)
  Reinterpret12/1 -> pipeline55/1(d)
  pipeline25/1(q) -> ab_re1/2(data_in)
  Reinterpret11/1 -> pipeline25/1(d)
  simple_bram_vacc9/2(valid) -> brams_control5/1(valid)
  Reinterpret14/1 -> pipeline23/1(d)
  brams_control5/1(we) -> ab_im0/3(we)
  pipeline23/1(q) -> ab_im0/2(data_in)
  pipeline62/1(q) -> ab_im0/1(addr)
  pipeline21/1(q) -> ab_re0/1(addr)
  simple_bram_vacc9/1(dout) -> Reinterpret14/1
  c_to_ri/1 -> Delay36/1
  simple_bram_vacc11/1(dout) -> Reinterpret12/1
  ab_re1/1(data_out) -> Terminator20/1
  simple_bram_vacc10/1(dout) -> Reinterpret11/1
  simple_bram_vacc8/2(valid) -> brams_control4/1(valid)
  c_to_ri/2 -> Delay37/1
  c_to_ri1/1 -> Delay39/1
  pipeline61/1(q) -> ab_re0/2(data_in)
  brams_control4/1(we) -> ab_re0/3(we)
  ab_im1/1(data_out) -> Terminator21/1
  Reinterpret13/1 -> pipeline61/1(d)
  ab_re0/1(data_out) -> Terminator18/1
  spect_cnt/1 -> pipeline1/1(d), pipeline7/1(d), pipeline18/1(d), pipeline20/1(d), pipeline21/1(d), pipeline62/1(d), pipeline26/1(d), pipeline56/1(d), pipeline63/1(d), pipeline71/1(d), pipeline66/1(d), pipeline69/1(d)
  simple_bram_vacc8/1(dout) -> Reinterpret13/1
  c_to_ri1/2 -> Delay40/1
  ab_im0/1(data_out) -> Terminator19/1
  ab_im2/1(data_out) -> Terminator23/1
  c_to_ri3/2 -> Delay42/1
  simple_bram_vacc14/1(dout) -> Reinterpret17/1
  ab_re2/1(data_out) -> Terminator22/1
  Reinterpret17/1 -> pipeline70/1(d)
  ab_im3/1(data_out) -> Terminator25/1
  brams_control8/1(we) -> ab_re2/3(we)
  pipeline70/1(q) -> ab_re2/2(data_in)
  c_to_ri3/1 -> Delay41/1
  c_to_ri2/2 -> Delay38/1
  simple_bram_vacc14/2(valid) -> brams_control8/1(valid)
  simple_bram_vacc12/1(dout) -> Reinterpret15/1
  ab_re3/1(data_out) -> Terminator24/1
  simple_bram_vacc13/1(dout) -> Reinterpret16/1
  c_to_ri2/1 -> Delay35/1
  simple_bram_vacc15/1(dout) -> Reinterpret18/1
  pipeline63/1(q) -> ab_re2/1(addr)
  pipeline71/1(q) -> ab_im2/1(addr)
  pipeline64/1(q) -> ab_im2/2(data_in)
  brams_control9/1(we) -> ab_im2/3(we)
  Reinterpret18/1 -> pipeline64/1(d)
  simple_bram_vacc15/2(valid) -> brams_control9/1(valid)
  Reinterpret15/1 -> pipeline65/1(d)
  pipeline65/1(q) -> ab_re3/2(data_in)
  Reinterpret16/1 -> pipeline68/1(d)
  pipeline69/1(q) -> ab_im3/1(addr)
  pipeline68/1(q) -> ab_im3/2(data_in)
  brams_control11/1(we) -> ab_im3/3(we)
  simple_bram_vacc13/2(valid) -> brams_control11/1(valid)
  simple_bram_vacc12/2(valid) -> brams_control10/1(valid)
  pipeline66/1(q) -> ab_re3/1(addr)
  brams_control10/1(we) -> ab_re3/3(we)
  pipeline100/1(q) -> simple_bram_vacc14/1(new_acc)
  pipeline101/1(q) -> simple_bram_vacc14/2(din)
  pipeline80/1(q) -> simple_bram_vacc15/1(new_acc)
  pipeline81/1(q) -> simple_bram_vacc15/2(din)
  pipeline90/1(q) -> simple_bram_vacc12/1(new_acc)
  pipeline95/1(q) -> simple_bram_vacc12/2(din)
  pipeline82/1(q) -> simple_bram_vacc13/1(new_acc)
  pipeline83/1(q) -> simple_bram_vacc13/2(din)
  pipeline96/1(q) -> pipeline101/1(d)
  pipeline97/1(q) -> pipeline81/1(d)
  pipeline98/1(q) -> pipeline95/1(d)
  pipeline99/1(q) -> pipeline83/1(d)
  Delay35/1 -> pipeline96/1(d)
  Delay38/1 -> pipeline97/1(d)
  Delay41/1 -> pipeline98/1(d)
  Delay42/1 -> pipeline99/1(d)
  cmult/1(ab) -> c_to_ri/1
  cmult1/1(ab) -> c_to_ri1/1
  cmult3/1(ab) -> c_to_ri3/1
  cmult2/1(ab) -> c_to_ri2/1
  [acc_len] -> acc_cntrl2/1(acc_len)
  pipeline113/1(q) -> spect_cnt2/1
  synth0_1/1(data_out) -> Terminator27/1
  power12/1 -> Delay48/1
  simple_bram_vacc16/1(dout) -> Reinterpret21/1
  spect_cnt2/1 -> pipeline43/1(d), pipeline86/1(d), pipeline84/1(d), pipeline54/1(d)
  synth0_0/1(data_out) -> Terminator26/1
  Reinterpret21/1 -> pipeline85/1(d)
  synth0_3/1(data_out) -> Terminator29/1
  brams_control16/1(we) -> synth0_0/3(we)
  pipeline85/1(q) -> synth0_0/2(data_in)
  power11/1 -> Delay47/1
  power10/1 -> Delay45/1
  simple_bram_vacc16/2(valid) -> brams_control16/1(valid), spect_cnt2/2
  simple_bram_vacc18/1(dout) -> Reinterpret19/1
  Delay43/1 -> acc_cntrl2/2(sync)
  synth0_2/1(data_out) -> Terminator28/1
  Delay46/1 -> Delay43/1
  [cnt_rst] -> acc_cntrl2/3(rst)
  simple_bram_vacc19/1(dout) -> Reinterpret20/1
  power9/1 -> Delay44/1
  simple_bram_vacc17/1(dout) -> Reinterpret22/1
  pipeline43/1(q) -> synth0_0/1(addr)
  pipeline86/1(q) -> synth0_1/1(addr)
  pipeline44/1(q) -> synth0_1/2(data_in)
  brams_control17/1(we) -> synth0_1/3(we)
  Reinterpret22/1 -> pipeline44/1(d)
  simple_bram_vacc17/2(valid) -> brams_control17/1(valid)
  Reinterpret19/1 -> pipeline45/1(d)
  pipeline45/1(q) -> synth0_2/2(data_in)
  Reinterpret20/1 -> pipeline79/1(d)
  pipeline84/1(q) -> synth0_3/1(addr)
  pipeline79/1(q) -> synth0_3/2(data_in)
  brams_control19/1(we) -> synth0_3/3(we)
  simple_bram_vacc19/2(valid) -> brams_control19/1(valid)
  simple_bram_vacc18/2(valid) -> brams_control18/1(valid)
  pipeline54/1(q) -> synth0_2/1(addr)
  brams_control18/1(we) -> synth0_2/3(we)
  pipeline125/1(q) -> simple_bram_vacc16/1(new_acc)
  pipeline126/1(q) -> simple_bram_vacc16/2(din)
  pipeline103/1(q) -> simple_bram_vacc17/1(new_acc)
  pipeline105/1(q) -> simple_bram_vacc17/2(din)
  pipeline115/1(q) -> simple_bram_vacc18/1(new_acc)
  pipeline120/1(q) -> simple_bram_vacc18/2(din)
  pipeline106/1(q) -> simple_bram_vacc19/1(new_acc)
  pipeline107/1(q) -> simple_bram_vacc19/2(din)
  acc_cntrl2/1(new_acc) -> pipeline113/1(d), pipeline106/1(d), pipeline115/1(d), pipeline103/1(d), pipeline125/1(d)
  pipeline121/1(q) -> pipeline126/1(d)
  pipeline122/1(q) -> pipeline105/1(d)
  pipeline123/1(q) -> pipeline120/1(d)
  pipeline124/1(q) -> pipeline107/1(d)
  Delay44/1 -> pipeline121/1(d)
  Delay45/1 -> pipeline122/1(d)
  Delay47/1 -> pipeline123/1(d)
  Delay48/1 -> pipeline124/1(d)
  Delay54/1 -> pipeline149/1(d)
  Delay53/1 -> pipeline148/1(d)
  Delay51/1 -> pipeline147/1(d)
  Delay50/1 -> pipeline146/1(d)
  pipeline149/1(q) -> pipeline132/1(d)
  pipeline148/1(q) -> pipeline145/1(d)
  pipeline147/1(q) -> pipeline130/1(d)
  pipeline146/1(q) -> pipeline151/1(d)
  acc_cntrl3/1(new_acc) -> pipeline150/1(d), pipeline128/1(d), pipeline140/1(d), pipeline131/1(d), pipeline138/1(d)
  pipeline132/1(q) -> simple_bram_vacc23/2(din)
  pipeline131/1(q) -> simple_bram_vacc23/1(new_acc)
  pipeline145/1(q) -> simple_bram_vacc22/2(din)
  pipeline140/1(q) -> simple_bram_vacc22/1(new_acc)
  pipeline128/1(q) -> simple_bram_vacc21/1(new_acc)
  pipeline151/1(q) -> simple_bram_vacc20/2(din)
  pipeline150/1(q) -> simple_bram_vacc20/1(new_acc)
  brams_control22/1(we) -> synth1_2/3(we)
  pipeline91/1(q) -> synth1_2/1(addr)
  simple_bram_vacc22/2(valid) -> brams_control22/1(valid)
  simple_bram_vacc23/2(valid) -> brams_control23/1(valid)
  brams_control23/1(we) -> synth1_3/3(we)
  pipeline92/1(q) -> synth1_3/2(data_in)
  pipeline93/1(q) -> synth1_3/1(addr)
  Reinterpret24/1 -> pipeline92/1(d)
  pipeline89/1(q) -> synth1_2/2(data_in)
  Reinterpret23/1 -> pipeline89/1(d)
  simple_bram_vacc21/2(valid) -> brams_control21/1(valid)
  Reinterpret26/1 -> pipeline88/1(d)
  brams_control21/1(we) -> synth1_1/3(we)
  pipeline88/1(q) -> synth1_1/2(data_in)
  pipeline102/1(q) -> synth1_1/1(addr)
  pipeline87/1(q) -> synth1_0/1(addr)
  simple_bram_vacc21/1(dout) -> Reinterpret26/1
  power16/1 -> Delay50/1
  simple_bram_vacc23/1(dout) -> Reinterpret24/1
  [cnt_rst] -> acc_cntrl3/3(rst)
  Delay52/1 -> Delay49/1
  synth1_2/1(data_out) -> Terminator32/1
  Delay49/1 -> acc_cntrl3/2(sync)
  simple_bram_vacc22/1(dout) -> Reinterpret23/1
  simple_bram_vacc20/2(valid) -> brams_control20/1(valid), spect_cnt3/2
  power13/1 -> Delay51/1
  power14/1 -> Delay53/1
  pipeline94/1(q) -> synth1_0/2(data_in)
  brams_control20/1(we) -> synth1_0/3(we)
  synth1_3/1(data_out) -> Terminator33/1
  Reinterpret25/1 -> pipeline94/1(d)
  synth1_0/1(data_out) -> Terminator30/1
  spect_cnt3/1 -> pipeline87/1(d), pipeline102/1(d), pipeline93/1(d), pipeline91/1(d)
  simple_bram_vacc20/1(dout) -> Reinterpret25/1
  power15/1 -> Delay54/1
  synth1_1/1(data_out) -> Terminator31/1
  pipeline138/1(q) -> spect_cnt3/1
  [acc_len] -> acc_cntrl3/1(acc_len)
  comp_add4/1(a+b) -> power16/1
  comp_add5/1(a+b) -> power13/1
  comp_add6/1(a+b) -> power14/1
  comp_add7/1(a+b) -> power15/1
  bram_mult1_0/1(c) -> comp_add4/2(b)
  pipeline153/1(q) -> comp_add4/1(a)
  bram_mult1_1/1(c) -> comp_add5/2(b)
  pipeline154/1(q) -> comp_add5/1(a)
  pipeline156/1(q) -> comp_add7/1(a)
  bram_mult1_3/1(c) -> comp_add7/2(b)
  pipeline155/1(q) -> comp_add6/1(a)
  bram_mult1_2/1(c) -> comp_add6/2(b)
  bram_mult0_2/1(c) -> comp_add2/2(b)
  pipeline159/1(q) -> comp_add2/1(a)
  bram_mult0_3/1(c) -> comp_add3/2(b)
  pipeline160/1(q) -> comp_add3/1(a)
  pipeline158/1(q) -> comp_add1/1(a)
  bram_mult0_1/1(c) -> comp_add1/2(b)
  pipeline157/1(q) -> comp_add/1(a)
  bram_mult0_0/1(c) -> comp_add/2(b)
  comp_add3/1(a+b) -> power12/1
  comp_add2/1(a+b) -> power11/1
  comp_add1/1(a+b) -> power10/1
  comp_add/1(a+b) -> power9/1
  pipeline161/1(q) -> Delay46/1
  pipeline162/1(q) -> pipeline161/1(d)
  pipeline164/1(q) -> pipeline163/1(d)
  pipeline163/1(q) -> Delay52/1
  Delay5/1 -> power1/1, bram_mult1_0/2(d), pipeline157/1(d), cmult/1(a)
  Delay17/1 -> power2/1, cmult1/1(a), bram_mult1_1/2(d), pipeline158/1(d)
  Delay3/1 -> power3/1, cmult2/1(a), bram_mult1_2/2(d), pipeline159/1(d)
  Delay7/1 -> power4/1, cmult3/1(a), bram_mult1_3/2(d), pipeline160/1(d)
  pipeline130/1(q) -> simple_bram_vacc21/2(din)
  write_brams/1(in_reg) -> pipeline165/1(d)
  pipeline165/1(q) -> [write_brams]
  Constant4/1 -> write_brams/1(sim_1)
